simulink model "r_128w_1k_1a_8x_r429"  (Simulink 7.2)
settings: Solver=FixedStepDiscrete  SolverMode=SingleTasking  StartTime=0.0  StopTime=5000  RelTol=1e-3
undefined symbols (not defined in the file): Down, pfb_coeff_gen_calc, Up
libraries: xbsIndex_r4 x8497, casper_library_misc x233, casper_library_delays x150, xps_library x70, simulink x28, dspsigops x12, casper_library_flow_control x8, casper_library_multipliers x8, xbsReplacements_r4 x4, casper_library_ffts_twiddle x2
(part 42 of 112)

== system r_128w_1k_1a_8x_r429/fft_wideband_real0/fft_direct/butterfly1_0/twiddle_general_4mult ==
  a : Inport
  b : Inport  Port=2
  sync : Inport  Port=3
  AddSub : xbsIndex_r4/AddSub  mode=Subtraction  use_carryin=off  use_carryout=off  en=off  latency=add_latency  precision=Full  arith_type=Unsigned  n_bits=16  bin_pt=14  quantization=Truncate  overflow=Wrap  dbl_ovrd=off  use_behavioral_HDL=on  hw_selection=Fabric  pipelined=off  block_type=addsub
  AddSub1 : xbsIndex_r4/AddSub  mode=Addition  use_carryin=off  use_carryout=off  en=off  latency=add_latency  precision=Full  arith_type=Unsigned  n_bits=16  bin_pt=14  quantization=Truncate  overflow=Wrap  dbl_ovrd=off  use_behavioral_HDL=on  hw_selection=Fabric  pipelined=off  block_type=addsub
  c_to_ri1 : casper_library_misc/c_to_ri  SystemSampleTime=-1  n_bits=input_bit_width  bin_pt=input_bit_width-1
      settings: AttributesFormatString=_ r/i
  c_to_ri2 : casper_library_misc/c_to_ri  SystemSampleTime=-1  n_bits=coeff_bit_width  bin_pt=coeff_bit_width-2
      settings: AttributesFormatString=_ r/i
  c_to_ri3 : casper_library_misc/c_to_ri  SystemSampleTime=-1  n_bits=input_bit_width  bin_pt=input_bit_width-1
      settings: AttributesFormatString=_ r/i
  coeff_gen : Subsystem  AncestorBlock=casper_library_ffts_twiddle_coeff_gen/coeff_gen  mask(coeff_gen){Coeffs=[1+0i       0.99998117528260110908888691483298-0.0061358846491544752690949771078976i        0.99992470183914450299056397852837-0.012271538285719925387429185548172i        0.99983058179582340319058175737155-0.018406729905804820185410974886508i        0.99969881869620424996725205346593-0.024541228522912288123603019585062i        0.99952941750109314256178549840115-0.030674803176636625950957082409332i        0.99932238458834954375475945198559-0.036807222941358831713731802892653i        0.99907772775264536146977434327709-0.042938256934940820241930481415693i        0.99879545620517240500646494183457-0.049067674327418014934565348994511i        0.99847558057329477421149022120517-0.055195244349689941143477511786841i        0.99811811290014917918966830256977-0.061320736302208578294425933563616i           0.9977230666441916362430220033275-0.0674439195636640509423642697584i        0.99729045667869020697082760307239-0.073564563599667426307959772202594i        0.99682029929116566790980868972838-0.079682437971430125633887087133189i          0.996312612182778001290728298045-0.085797312344439893849745715215249i        0.99576741446765981713440396561055-0.091908956497132723861831493650243i        0.99518472667219692873175063141389-0.098017140329560603628777926132898i         0.99456457073425541537403660186101-0.10412163387205458642270627933613i         0.99390697000235606051177228437155-0.11022220729388305937579417559391i         0.99321194923479450000769475082052-0.11631863091190475234526502390509i         0.99247953459870996706371215623221-0.12241067519921619566325432515441i         0.99170975366909952519733906228794-0.12849811079379316880455519367388i         0.99090263542778000971367191596073-0.13458070850712616772781871077314i          0.99005821026229712256139237069874-0.1406582393328492108786065273307i         0.98917650996478101443898367506335-0.14673047445536174793190298260015i         0.98825756773074946437418475397862-0.15279718525844343535169400638551i         0.98730141815785843473207705756067-0.15885814333386144570070541703899i         0.98630809724459866938417462733923-0.16491312048996992212224199647608i         0.98527764238894122161838140527834-0.17096188876030121717164433903235i         0.98421009238692902520995176018914-0.17700422041214874946390978038835i         0.98310548743121628501029363178532-0.18303988795514095078154070961318i         0.98196386910955524296440444231848-0.18906866414980619262031780181132i         0.98078528040323043057924223830923-0.19509032201612824808378832130984i         0.97956976568544051886533452488948-0.20110463484209190054841087658133i         0.97831737071962765472932233024039-0.20711137619221856032147854875802i         0.97702814265775439483974196264171-0.21311031991609136193588369678764i         0.97570213003852857003295184767921-0.21910124015686979759287567048887i          0.97433938278557585821459952057921-0.2250839113597928320409380376077i          0.9729399522055601767789312361856-0.23105810828067110951344886871084i         0.97150389098625178352364173406386-0.23702360599436719801325068601727i         0.97003125319454397423868385885726-0.24298017990326387094413007616822i         0.96852209427441726674601341073867-0.24892760574572014853345081064617i         0.96697647104485207059099138859892-0.25486565960451457169000377689372i         0.96539444169768939829623377590906-0.26079411791527551400804441072978i         0.96377606579543984022251379428781-0.26671275747489836538406393628975i         0.96212140426904158019283386238385-0.27262135544994897662363086965343i         0.96043051941556578654513032233808-0.27851968938505305972697101424274i         0.95870347489587159905966018413892-0.28440753721127187692019333553617i         0.95694033573220882438192802510457-0.29028467725446233105301985233382i         0.95514116830577078243180721983663-0.29615088824362378883137125740177i          0.95330604035419386210747916265973-0.3020059493192280841711294669949i ...(+36411 chars)], coeff_bit_width=coeff_bit_width, StepPeriod=0, bram_latency=bram_latency, coeffs_bram=on}
      mask prompts: Coeffs "Coefficients: (0 to 2^(FFTSize-1)-1)"; coeff_bit_width "Coefficient Bit Width:"; StepPeriod "Step Period: (2^?)"; bram_latency "Latency:"; coeffs_bram "Store coefficients in BRAM"
      mask help: Generates a sequence of complex coefficients
      mask init:
      | coeff_gen_init( gcb, ...
      |     'Coeffs', Coeffs, ...
      |     'coeff_bit_width', coeff_bit_width, ...
      |     'StepPeriod', StepPeriod, ...
      |     'bram_latency', bram_latency, ...
      |     'coeffs_bram', coeffs_bram);
      settings: AttributesFormatString=512 @ (18,16)
      mask CallbackString:
      | Coeffs = eval(get_param(gcb, 'Coeffs'));
      | if( length(Coeffs) == 1 ),
      |     set_param(gcb, 'MaskVisibilities',{'on','on','off','off','off'});
      | else
      |     set_param(gcb, 'MaskVisibilities',{'on','on','on','on','on'});
      | end||||
  convert0 : xbsIndex_r4/Convert  arith_type="Signed  (2's comp)"  n_bits=input_bit_width+4  bin_pt=input_bit_width+1  quantization="Round  (unbiased: Even Values)"  overflow=Wrap  latency=conv_latency  dbl_ovrd=off  pipeline=on  block_type=convert
      settings: block_version=11.4
  convert1 : xbsIndex_r4/Convert  arith_type="Signed  (2's comp)"  n_bits=input_bit_width+4  bin_pt=input_bit_width+1  quantization="Round  (unbiased: Even Values)"  overflow=Wrap  latency=conv_latency  dbl_ovrd=off  pipeline=on  block_type=convert
      settings: block_version=11.4
  delay0 : xbsIndex_r4/Delay  en=off  latency=mult_latency + add_latency + bram_latency + conv_latency  dbl_ovrd=off  reg_retiming=off  block_type=delay
      settings: block_version=11.4
  delay1 : xbsIndex_r4/Delay  en=off  latency=bram_latency  dbl_ovrd=off  reg_retiming=on  block_type=delay
      settings: block_version=11.4
  delay2 : xbsIndex_r4/Delay  en=off  latency=mult_latency + add_latency + bram_latency + conv_latency  dbl_ovrd=off  reg_retiming=off  block_type=delay
      settings: block_version=11.4
  mult{,1..3} (4 alike) : xbsIndex_r4/Mult  precision=Full  arith_type="Signed  (2's comp)"  n_bits=16  bin_pt=14  quantization=Truncate  overflow=Wrap  en=off  latency=mult_latency  dbl_ovrd=off  use_behavioral_HDL=on  opt=Speed  use_embedded=off  optimum_pipeline=off  pipeline=on  use_rpm=on  placement_style=Triangular  block_type=mult
      settings: block_version=11.4
  a_re : Outport
  a_im : Outport  Port=2
  bw_re : Outport  Port=3
  bw_im : Outport  Port=4
  sync_out : Outport  Port=5
  wires:
  a/1 -> delay0/1
  delay0/1 -> c_to_ri1/1
  c_to_ri1/1 -> a_re/1
  c_to_ri1/2 -> a_im/1
  sync/1 -> delay2/1, coeff_gen/1(rst)
  delay2/1 -> sync_out/1
  coeff_gen/1(w) -> c_to_ri2/1
  b/1 -> delay1/1
  delay1/1 -> c_to_ri3/1
  c_to_ri3/1 -> mult/1, mult3/1
  c_to_ri2/1 -> mult/2, mult1/2
  c_to_ri3/2 -> mult1/1, mult2/1
  c_to_ri2/2 -> mult2/2, mult3/2
  mult/1 -> AddSub/1
  mult1/1 -> AddSub1/1
  mult2/1 -> AddSub/2
  mult3/1 -> AddSub1/2
  AddSub/1 -> convert0/1
  convert0/1 -> bw_re/1
  AddSub1/1 -> convert1/1
  convert1/1 -> bw_im/1
